simulink model "MCVT_Integration"  (R2020a)
settings: Solver=ode8  StartTime=0.0  StopTime=T_end  FixedStep=dt  RelTol=1e-3  EnableMultiTasking=on
values from neighbouring files (symbols this model uses):
  assumed_power_voltage = 1.25e2    (= 125)    [MCVT_Input_File.m]    (also in MCVT_Run_QuickCheck.m)
  battery_power_toggle = 1    [MCVT_Input_File.m]    (also in MCVT_Run_QuickCheck.m)
  battery_trickle_charge_param = 0.1    [MCVT_Input_File.m]    (also in MCVT_Run_QuickCheck.m)
  battery_trickle_leakage_param = 0.01    [MCVT_Input_File.m]    (also in MCVT_Run_QuickCheck.m)
  damage_case_id = 3    [MCVT_Input_File.m]    (also in MCVT_Run_QuickCheck.m = 1)
  dt = 1/fs    (= 0.001)    [MCVT_Run_QuickCheck.m]    (also in MCVT_Sim_Set.m)
  dt_2 = 1/fs_2    (= 0.001)    [MCVT_Run_QuickCheck.m]    (also in MCVT_Sim_Set.m)
  dt_3 = 1/fs_3    (= 0.001)    [MCVT_Run_QuickCheck.m]    (also in MCVT_Sim_Set.m)
  dt_5 = 1/fs_5    (= 0.005)    [MCVT_Run_QuickCheck.m]    (also in MCVT_Sim_Set.m)
  dt_8 = 1/fs_8    (= 0.05)    [MCVT_Run_QuickCheck.m]    (also in MCVT_Sim_Set.m)
  dt_9 = 1/fs_9    (= 0.001)    [MCVT_Run_QuickCheck.m]    (also in MCVT_Sim_Set.m)
  dust_met_duration = 3    [MCVT_Input_File.m]    (also in MCVT_Run_QuickCheck.m = 2)
  ECLSS_indoor_set_temperature = 298.15    [MCVT_Input_File.m]    (also in MCVT_Run_QuickCheck.m)
  energy_store_max_o = 150    [MCVT_Input_File.m]    (also in MCVT_Run_QuickCheck.m)
  energy_store_o = 120    [MCVT_Input_File.m]    (also in MCVT_Run_QuickCheck.m)
  fs = 1000    [MCVT_Run_QuickCheck.m]    (also in MCVT_Sim_Set.m)
  gain_charge_param = 1    [MCVT_Input_File.m]    (also in MCVT_Run_QuickCheck.m)
  ground_temperature = 373    [MCVT_Habitat_Design_Input_File.m]    (also in MCVT_Run_QuickCheck.m)
  I_max_charge = 800    [MCVT_Input_File.m]    (also in MCVT_Run_QuickCheck.m)
  I_max_discharge = 800    [MCVT_Input_File.m]    (also in MCVT_Run_QuickCheck.m)
  initial_pressure = 1e5    (= 100000)    [MCVT_Input_File.m]    (also in MCVT_Run_QuickCheck.m)
  initial_temperature = 295    [MCVT_Input_File.m]    (also in MCVT_Run_QuickCheck.m)
  interior_leak_rate = 0.00415    [MCVT_Input_File.m]    (also in MCVT_Run_QuickCheck.m)
  launch_dust_accum_start_time = 10    [MCVT_Input_File.m]    (also in MCVT_Run_QuickCheck.m = 2)
  launch_dust_accum_stop_time = 12    [MCVT_Input_File.m]    (also in MCVT_Run_QuickCheck.m = 3)
  meteorite_impact_time = 2    [MCVT_Input_File.m]    (also in MCVT_Run_QuickCheck.m = 1)
  nuclear_power_toggle = 1    [MCVT_Input_File.m]    (also in MCVT_Run_QuickCheck.m)
  print_interval = 30    [MCVT_Input_File.m]
  SOC_lower_thres = 0.5    [MCVT_Input_File.m]    (also in MCVT_Run_QuickCheck.m)
  SOC_upper_thres = 0.9    [MCVT_Input_File.m]    (also in MCVT_Run_QuickCheck.m)
  solar_power_toggle = 1    [MCVT_Input_File.m]    (also in MCVT_Run_QuickCheck.m)
  space_temperature = 4    [MCVT_Habitat_Design_Input_File.m]    (also in MCVT_Run_QuickCheck.m)
  Start_angle = 90    [MCVT_Input_File.m]    (also in MCVT_Run_QuickCheck.m)
undefined symbols (not defined in the file or in its folder): A_flow_restrictor, ACTIVITY_LOOKUP_TABLE, air, Aref, Bmap, Bmap2, Bmap3, comms, DiscIntNeverNeededParam, DmgSPL, dust_parameters, ECLSS_dust_damage_repair_time, ECLSS_heater_capacity, ECLSS_IPS_restrictor, ECLSS_IPS_setpointPressure, ECLSS_paint_damage_repair_time, ECLSS_radiatorLoop_discreteNumber, ECLSS_Vst, emptyActivity, expected_heat_add, FI, habitat, HIEM2SMM, HIEM2STM, HIEMDamageElements, impact_numbering, initial_SPLT_damage_index, input_air, input_Cond, input_CP, input_discretization, input_EV, input_Evap, input_radiatorLoop, K_ECLSS_dust_single, K_ECLSS_paint_single, K_Structure_damage_single, Kref, kW_to_W, meteoroidDustDep, mFRF, ndelays, nominalDustDep, noverlap, nuclear_toggle_gain, output_limits, panel_paint0, Ps0, reference_temperature, RepairTime_ECLSS_factor, Sensor_Location_SMM, SMM_T0, SMM_T1, SMM_T2, SMM_T3, SMM_T4, SMM_T4_0, SMM_T4_1, SMM_T4_2, solar_FDD_K ... (+73)
libraries: neural x350, simulink x39, xpcutilitieslib x7, xpcrtudplib x7, dspstat3 x1
(part 31 of 43)

== system MCVT_Integration/Sys2 - Structural System/Sys2_Structural_System/Fault Detection and Diagnosis (FDD) with Binary Classifer/Pattern Recognition Neural Network/Segment 5/Layer 2/Delays 1 ==
  "p{2}" : Inport  PortDimensions=20
      mask Display: e = 0.06;c1 = [120 230 180]/255;c2 = ([120 230 180]+0)/(256+128);c3 = ([120 230 180]+128)/(256+128);patch([0 1 1 0],[0 0 1 1],c1);patch([0 1 1 1-e 1-e 0+e],[0 0 1 1-e 0+e 0+e],c2);patch([0 0 1 1-e 0+e 0+e],[0 1 1 1-e 1-e 0+e],c3);plot([0 1 1 0 0],[0 0 1 1 0]);
  mux : Mux  Inputs=1
      mask Display: e = 0.06;c1 = [120 150 230]/255;c2 = ([120 150 230]+0)/383;c3 = ([120 150 230]+128)/383;patch([0 1 1 0],[0 0 1 1],c1);patch([0 1 1 1-e 1-e 0+e],[0 0 1 1-e 0+e 0+e],c2);patch([0 0 1 1-e 0+e 0+e],[0 1 1 1-e 1-e 0+e],c3);plot([0 1 1 0 0],[0 0 1 1 0]);disp('Mux')
  "pd{2,1}" : Outport
      mask Display: e = 0.06;c1 = [120 230 180]/255;c2 = ([120 230 180]+0)/(256+128);c3 = ([120 230 180]+128)/(256+128);patch([0 1 1 0],[0 0 1 1],c1);patch([0 1 1 1-e 1-e 0+e],[0 0 1 1-e 0+e 0+e],c2);patch([0 0 1 1-e 0+e 0+e],[0 1 1 1-e 1-e 0+e],c3);plot([0 1 1 0 0],[0 0 1 1 0]);
  wires:
  "p{2}"/1 -> mux/1
  mux/1 -> "pd{2,1}"/1

== system MCVT_Integration/Sys2 - Structural System/Sys2_Structural_System/Fault Detection and Diagnosis (FDD) with Binary Classifer/Pattern Recognition Neural Network/Segment 5/Layer 2/LW{2,1} ==
  "ad{2,1}" : Inport  PortDimensions=20
      mask Display: e = 0.06;c1 = [120 230 180]/255;c2 = ([120 230 180]+0)/(256+128);c3 = ([120 230 180]+128)/(256+128);patch([0 1 1 0],[0 0 1 1],c1);patch([0 1 1 1-e 1-e 0+e],[0 0 1 1-e 0+e 0+e],c2);patch([0 0 1 1-e 0+e 0+e],[0 1 1 1-e 1-e 0+e],c3);plot([0 1 1 0 0],[0 0 1 1 0]);
  "IW{2,1}(1,:)'" : Constant  Value=[-0.67773218447365179972763371551991440355777740478515625;0.29723131391808177426128168008290231227874755859375;-0.09310950608786626447876955126048414967954158782958984375;-0.25043409990960252553549025833490304648876190185546875;0.61520314771236161988099411246366798877716064453125;-0.4413810227549539444424908651853911578655242919921875;0.34640993668388009307790298407780937850475311279296875;0.58842174257296786521465037367306649684906005859375;-0.5081607293297121241693048432352952659130096435546875;0.033421296078495636716620964534740778617560863494873046875;0.496988773917953319259055433576577343046665191650390625;0.2455390127671049083080134778356296010315418243408203125;-0.224996153594978764989065211921115405857563018798828125;-0.3087985696736519347638250110321678221225738525390625;0.4009273835608577751798975441488437354564666748046875;-0.2431356717879919748792616474020178429782390594482421875;-0.00285707632105562832347533941401707124896347522735595703125;0.032728987946543490472617321529469336383044719696044921875;0.5245186178259164666570768531528301537036895751953125;-0.1546591117435880680108795104388264007866382598876953125]
      mask Display: e = 0.06;c1 = [120 150 230]/255;c2 = ([120 150 230]+0)/383;c3 = ([120 150 230]+128)/383;patch([0 1 1 0],[0 0 1 1],c1);patch([0 1 1 1-e 1-e 0+e],[0 0 1 1-e 0+e 0+e],c2);patch([0 0 1 1-e 0+e 0+e],[0 1 1 1-e 1-e 0+e],c3);plot([0 1 1 0 0],[0 0 1 1 0]);disp('weights');
  "IW{2,1}(10,:)'" : Constant  Value=[0.552549033571831937905471932026557624340057373046875;-0.09100490165966072797498753743639099411666393280029296875;0.1760541628457268081842101992151583544909954071044921875;0.5874239097493589856213702660170383751392364501953125;0.130139051658360982433038088856847025454044342041015625;0.5921645595936595452002393358270637691020965576171875;-0.06521020054191943582377888333212467841804027557373046875;0.28897880348058435640012930889497511088848114013671875;-0.1298384734383723204675931128804222680628299713134765625;-0.314572028887444898881398103185347281396389007568359375;0.65930167336349521089999825562699697911739349365234375;0.237364684824766303261611710695433430373668670654296875;-0.054096999788288357979837428501923568546772003173828125;0.51311436402968946257630022955709137022495269775390625;-0.55296838715594442970058253195020370185375213623046875;-0.1727245527295988292149075959969195537269115447998046875;-0.10587060479695453718562703215866349637508392333984375;-0.283737366362507958950089914651471190154552459716796875;0.26502997291755281406011590661364607512950897216796875;0.281267240887428382478674393496476113796234130859375]
      mask Display: e = 0.06;c1 = [120 150 230]/255;c2 = ([120 150 230]+0)/383;c3 = ([120 150 230]+128)/383;patch([0 1 1 0],[0 0 1 1],c1);patch([0 1 1 1-e 1-e 0+e],[0 0 1 1-e 0+e 0+e],c2);patch([0 0 1 1-e 0+e 0+e],[0 1 1 1-e 1-e 0+e],c3);plot([0 1 1 0 0],[0 0 1 1 0]);disp('weights');
  "IW{2,1}(11,:)'" : Constant  Value=[-0.05912874150283971286246043064238619990646839141845703125;0.54156082763547741532050849855295382440090179443359375;0.1892905576102281439698771237090113572776317596435546875;0.266587539549432428476194445465807802975177764892578125;0.2437897926806707749047831157440668903291225433349609375;-0.360503628753871208090231448295526206493377685546875;0.38970268980417788196035644432413391768932342529296875;0.01812511205154222759805549003431224264204502105712890625;-0.51079805029018710005317416289472021162509918212890625;0.331332206449338972920060086835292167961597442626953125;0.5113925621810591071181306688231416046619415283203125;-0.0743364509739538326105190435555414296686649322509765625;-0.088415771036461532350614334063720889389514923095703125;-0.5054613948697108671836986104608513414859771728515625;0.56960695088877766334434227246674709022045135498046875;-0.235268335550750384843610163443372584879398345947265625;0.4062060320473663832530064610182307660579681396484375;-0.4762276658674691187655980684212408959865570068359375;0.385169708871014704332225164762348867952823638916015625;-0.277613748702794704836804839942487888038158416748046875]
      mask Display: e = 0.06;c1 = [120 150 230]/255;c2 = ([120 150 230]+0)/383;c3 = ([120 150 230]+128)/383;patch([0 1 1 0],[0 0 1 1],c1);patch([0 1 1 1-e 1-e 0+e],[0 0 1 1-e 0+e 0+e],c2);patch([0 0 1 1-e 0+e 0+e],[0 1 1 1-e 1-e 0+e],c3);plot([0 1 1 0 0],[0 0 1 1 0]);disp('weights');
  "IW{2,1}(12,:)'" : Constant  Value=[0.43773075430955421527556836736039258539676666259765625;-0.0226631485883267702607923865798511542379856109619140625;0.1375752179635638672294817297370173037052154541015625;-0.342589307385872621214417677037999965250492095947265625;-0.00803372039310569323322663848330194014124572277069091796875;0.266163547138629719146507568439119495451450347900390625;0.0679141773643662516324326361427665688097476959228515625;0.447036832445153742288113107861136086285114288330078125;0.338363274166047001134671745603554882109165191650390625;0.63438020299558761649905136437155306339263916015625;0.4849211604715624179817723415908403694629669189453125;0.2307920076735069658813159776400425471365451812744140625;0.641689290985569815717326491721905767917633056640625;-0.61592841776821616317505458937375806272029876708984375;0.482357229715567437100531833493732847273349761962890625;-0.0080342268978742213680899197925100452266633510589599609375;0.299017768098594538539458653758629225194454193115234375;0.1865716668674242129100093734450638294219970703125;0.152140058661927202177110984848695807158946990966796875;-0.09721810243248285576811440478195436298847198486328125]
      mask Display: e = 0.06;c1 = [120 150 230]/255;c2 = ([120 150 230]+0)/383;c3 = ([120 150 230]+128)/383;patch([0 1 1 0],[0 0 1 1],c1);patch([0 1 1 1-e 1-e 0+e],[0 0 1 1-e 0+e 0+e],c2);patch([0 0 1 1-e 0+e 0+e],[0 1 1 1-e 1-e 0+e],c3);plot([0 1 1 0 0],[0 0 1 1 0]);disp('weights');
  "IW{2,1}(13,:)'" : Constant  Value=[0.4355595750049321424057779950089752674102783203125;-0.0895990004445349208506144123020931147038936614990234375;-0.54399989225422562544309812437859363853931427001953125;-0.373034500974306515441725196069455705583095550537109375;0.11066112771720294782884508322240435518324375152587890625;-0.3757367253088403913352522067725658416748046875;0.499029093144363000700280963428667746484279632568359375;-0.106510080176182408973062365475925616919994354248046875;0.320524922721690141980133148535969667136669158935546875;-0.16644989950893285257649267805390991270542144775390625;-0.035674542064537760610587469045640318654477596282958984375;-0.418057933762240152564260142753482796251773834228515625;-0.388561217554914739569227322135702706873416900634765625;0.13753317886975857664566547100548632442951202392578125;0.211068009211963392157684893390978686511516571044921875;0.51951396420677331544624166781431995332241058349609375;0.56988508309968899379072126976097933948040008544921875;0.481247016510891578722208805629634298384189605712890625;0.44357156438096811967852772795595228672027587890625;0.255785238287536575985114950526622124016284942626953125]
      mask Display: e = 0.06;c1 = [120 150 230]/255;c2 = ([120 150 230]+0)/383;c3 = ([120 150 230]+128)/383;patch([0 1 1 0],[0 0 1 1],c1);patch([0 1 1 1-e 1-e 0+e],[0 0 1 1-e 0+e 0+e],c2);patch([0 0 1 1-e 0+e 0+e],[0 1 1 1-e 1-e 0+e],c3);plot([0 1 1 0 0],[0 0 1 1 0]);disp('weights');
  "IW{2,1}(14,:)'" : Constant  Value=[-0.039786612181184351222373862810854916460812091827392578125;0.2321373358663718911021334179167752154171466827392578125;0.08032148343902671683292027182687888853251934051513671875;0.66783349466954777806648735349881462752819061279296875;0.0218740404012538088018846593740818207152187824249267578125;-0.284151817373596349280973072382039390504360198974609375;0.406206696173917414238729861608589999377727508544921875;-0.05018628210290389246583941940116346813738346099853515625;-0.111863721242385250054240941608441062271595001220703125;-0.59457414197112290477065243976539932191371917724609375;-0.00855983743492590154477994701665011234581470489501953125;-0.053293368746645163047670479272710508666932582855224609375;0.35542157490681181020164558503893204033374786376953125;0.5503721492865896181001517106778919696807861328125;-0.293494096559414108238428298136568628251552581787109375;-0.379628681256232669571915039341547526419162750244140625;-0.236013920704870983069412204713444225490093231201171875;0.47230602229098217481606525325332768261432647705078125;0.09948027948272873677293404170995927415788173675537109375;0.55264058309173236249733918157289735972881317138671875]
      mask Display: e = 0.06;c1 = [120 150 230]/255;c2 = ([120 150 230]+0)/383;c3 = ([120 150 230]+128)/383;patch([0 1 1 0],[0 0 1 1],c1);patch([0 1 1 1-e 1-e 0+e],[0 0 1 1-e 0+e 0+e],c2);patch([0 0 1 1-e 0+e 0+e],[0 1 1 1-e 1-e 0+e],c3);plot([0 1 1 0 0],[0 0 1 1 0]);disp('weights');
  "IW{2,1}(15,:)'" : Constant  Value=[0.1288107674958688664279549129787483252584934234619140625;-0.07497256937682771071873588653033948503434658050537109375;-0.30959773506689558519866523056407459080219268798828125;0.448215012435883630725896864532842300832271575927734375;-0.361768940527965099374085866656969301402568817138671875;-0.54297214933572546247120271800667978823184967041015625;0.054457371666344657479275070954827242530882358551025390625;0.125959936202742095456841298073413781821727752685546875;-0.2056921734268315204996468992248992435634136199951171875;0.440254257234804213805290373784373514354228973388671875;0.51034921534097066331270298178424127399921417236328125;0.468177463702322882443951357345213182270526885986328125;0.65075745752746716465964027520385570824146270751953125;-0.12131802595037254055565512089742696844041347503662109375;-0.42844264696869771569254226051270961761474609375;-0.323720290416319833592950772072072140872478485107421875;-0.064098745923206135177707665206980891525745391845703125;0.63669387601472882121100838048732839524745941162109375;-0.052305465433853150225562700370574020780622959136962890625;0.05665559541268076759745753179231542162597179412841796875]
      mask Display: e = 0.06;c1 = [120 150 230]/255;c2 = ([120 150 230]+0)/383;c3 = ([120 150 230]+128)/383;patch([0 1 1 0],[0 0 1 1],c1);patch([0 1 1 1-e 1-e 0+e],[0 0 1 1-e 0+e 0+e],c2);patch([0 0 1 1-e 0+e 0+e],[0 1 1 1-e 1-e 0+e],c3);plot([0 1 1 0 0],[0 0 1 1 0]);disp('weights');
  "IW{2,1}(16,:)'" : Constant  Value=[0.11737245423700033708502843410315108485519886016845703125;-0.27262057499895442358450736719532869756221771240234375;-0.07361864460219173789123914275478455238044261932373046875;-0.29779470754941239629687288470449857413768768310546875;0.386666490528341511012655473678023554384708404541015625;0.2476893163085038540582871746664750389754772186279296875;-0.6814743935251723616630670221638865768909454345703125;-0.59199523499185369157515879123820923268795013427734375;-0.299505833684180544640440757575561292469501495361328125;0.214060293872676610238414696141262538731098175048828125;-0.462623005264232833866344662965275347232818603515625;0.319710052566719860056565494232927449047565460205078125;-0.2728474794043964646306221766280941665172576904296875;0.265651446928187373597296527805156074464321136474609375;0.65459409742826590861142221910995431244373321533203125;-0.31617548578397947078855168001609854400157928466796875;-0.4149790189200015522175135629368014633655548095703125;-0.216229979661193283391185104846954345703125;-0.0700132160782936108223140081463498063385486602783203125;-0.29381327313157579173008571160607971251010894775390625]
      mask Display: e = 0.06;c1 = [120 150 230]/255;c2 = ([120 150 230]+0)/383;c3 = ([120 150 230]+128)/383;patch([0 1 1 0],[0 0 1 1],c1);patch([0 1 1 1-e 1-e 0+e],[0 0 1 1-e 0+e 0+e],c2);patch([0 0 1 1-e 0+e 0+e],[0 1 1 1-e 1-e 0+e],c3);plot([0 1 1 0 0],[0 0 1 1 0]);disp('weights');
  "IW{2,1}(17,:)'" : Constant  Value=[-0.65200183797321809020530736233922652900218963623046875;-0.513141636890571906093327925191260874271392822265625;0.6666627640293854017983221638132818043231964111328125;0.51126666389086794506368960355757735669612884521484375;0.142832758061001763305597478392883203923702239990234375;0.0309716089851164409763040197276495746336877346038818359375;-0.51906860495920581488604739206493832170963287353515625;0.455849206301122855666818622921709902584552764892578125;-0.5074134051478431661763579541002400219440460205078125;-0.483029589404079473524689092300832271575927734375;0.224793030869896603984869898340548388659954071044921875;0.168491582461909483736661741204443387687206268310546875;0.49576084119203611688675437108031474053859710693359375;-0.0518882463700796880967658353256410919129848480224609375;-0.458851615048450067746443892247043550014495849609375;0.1592196075605500638072697938696364872157573699951171875;-0.393400321923802642753997815816546790301799774169921875;0.06488101432483096842052106012488366104662418365478515625;-0.08832697515481234551426581447231001220643520355224609375;-0.310794878933715990054764688466093502938747406005859375]
      mask Display: e = 0.06;c1 = [120 150 230]/255;c2 = ([120 150 230]+0)/383;c3 = ([120 150 230]+128)/383;patch([0 1 1 0],[0 0 1 1],c1);patch([0 1 1 1-e 1-e 0+e],[0 0 1 1-e 0+e 0+e],c2);patch([0 0 1 1-e 0+e 0+e],[0 1 1 1-e 1-e 0+e],c3);plot([0 1 1 0 0],[0 0 1 1 0]);disp('weights');
  "IW{2,1}(18,:)'" : Constant  Value=[-0.58403843431543833730756887234747409820556640625;-0.11686344353476274537939616493531502783298492431640625;-0.208421829485184562091859561405726708471775054931640625;0.350835116678501368614462307959911413490772247314453125;-0.250053018739082866250811321151559241116046905517578125;-0.1767589718090601114131033000376191921532154083251953125;-0.6594792324061919952526977795059792697429656982421875;-0.2047483842336772219550056206571753136813640594482421875;0.0799817324020934972139684759895317256450653076171875;-0.263873314132044234359142365065054036676883697509765625;0.1238058615990984823174159146219608373939990997314453125;-0.2427010547863654721378878775794873945415019989013671875;-0.340912365339598844027335644568665884435176849365234375;-0.40629502196977806960376256029121577739715576171875;0.05821085493265502897042296126528526656329631805419921875;-0.4923309925498069450355842491262592375278472900390625;-0.63697077823032144383574859602958895266056060791015625;0.70272777928235630184872206882573664188385009765625;0.082408225308850002743810136962565593421459197998046875;0.257126723858133787725677166235982440412044525146484375]
      mask Display: e = 0.06;c1 = [120 150 230]/255;c2 = ([120 150 230]+0)/383;c3 = ([120 150 230]+128)/383;patch([0 1 1 0],[0 0 1 1],c1);patch([0 1 1 1-e 1-e 0+e],[0 0 1 1-e 0+e 0+e],c2);patch([0 0 1 1-e 0+e 0+e],[0 1 1 1-e 1-e 0+e],c3);plot([0 1 1 0 0],[0 0 1 1 0]);disp('weights');
  "IW{2,1}(19,:)'" : Constant  Value=[0.66085390281812073087763792500481940805912017822265625;-0.4135361056779769395319590330473147332668304443359375;0.1428003055410909383216022661144961602985858917236328125;0.39212017801967302688126437715254724025726318359375;-0.5914533321008648147909525505383498966693878173828125;0.468354086242417422258910164600820280611515045166015625;0.33818694482531197476049555916688404977321624755859375;-0.10651793410114475391115007596454233862459659576416015625;0.01404438680674700845596447607022128067910671234130859375;-0.067871504435023599643983516216394491493701934814453125;-0.58546404149150454987449165855650790035724639892578125;-0.24986074727083884550182801831397227942943572998046875;-0.33108516389830178194841892036492936313152313232421875;0.1009772519076451990915899159517721273005008697509765625;-0.2363686013993024082058269641493097878992557525634765625;-0.62080475837335746813749892680789344012737274169921875;0.25979730822451652105797847980284132063388824462890625;-0.31588222351298089751736597463604994118213653564453125;-0.449657863213273056857133269659243524074554443359375;0.48004422581746719611572871144744567573070526123046875]
      mask Display: e = 0.06;c1 = [120 150 230]/255;c2 = ([120 150 230]+0)/383;c3 = ([120 150 230]+128)/383;patch([0 1 1 0],[0 0 1 1],c1);patch([0 1 1 1-e 1-e 0+e],[0 0 1 1-e 0+e 0+e],c2);patch([0 0 1 1-e 0+e 0+e],[0 1 1 1-e 1-e 0+e],c3);plot([0 1 1 0 0],[0 0 1 1 0]);disp('weights');
  "IW{2,1}(2,:)'" : Constant  Value=[0.264866509139968731023628834009286947548389434814453125;-0.421276202799477472726863425123156048357486724853515625;-0.32172884927020184431256666357512585818767547607421875;0.0873332852793348435316289624097407795488834381103515625;0.45725062710630892670593539151013828814029693603515625;-0.47027317103559440614191089480300433933734893798828125;0.50420051952175215514984074616222642362117767333984375;0.2021495935450947911693475589345325715839862823486328125;0.5958119042887479732684141708887182176113128662109375;0.549176439745886479926184620126150548458099365234375;-0.07382040642580724176813333770041936077177524566650390625;-0.0911008824706815711902407883826526813209056854248046875;0.472789640540149047609475019271485507488250732421875;-0.302487138214584894146952365190372802317142486572265625;0.13705931754781619513750001715379767119884490966796875;0.02691598569268426055511866934466524980962276458740234375;-0.61260682814079270297469292927416972815990447998046875;0.212242740676705288205283750357921235263347625732421875;-0.45586121041979865697868490315158851444721221923828125;-0.173654441390307334724951715543284080922603607177734375]
      mask Display: e = 0.06;c1 = [120 150 230]/255;c2 = ([120 150 230]+0)/383;c3 = ([120 150 230]+128)/383;patch([0 1 1 0],[0 0 1 1],c1);patch([0 1 1 1-e 1-e 0+e],[0 0 1 1-e 0+e 0+e],c2);patch([0 0 1 1-e 0+e 0+e],[0 1 1 1-e 1-e 0+e],c3);plot([0 1 1 0 0],[0 0 1 1 0]);disp('weights');
  "IW{2,1}(20,:)'" : Constant  Value=[-0.4175468971215832159060710182529874145984649658203125;0.03969986159828763072621171659193350933492183685302734375;-0.1566172687200387547701296853119856677949428558349609375;-0.32922271339706554726944887079298496246337890625;-0.51801946227475414996632707698154263198375701904296875;-0.307456855608206625074529938501655124127864837646484375;0.384731736291375525649272049122373573482036590576171875;0.04812249993952956439446921876879059709608554840087890625;-0.090638927458235374334805101170786656439304351806640625;0.122542975183939584216119556003832258284091949462890625;-0.29383378097437728992957772788940928876399993896484375;0.438785700581473037118485081009566783905029296875;-0.399251041064929090840252001726184971630573272705078125;0.35533599456226572232964144859579391777515411376953125;0.4899082557321496889102263594395481050014495849609375;-0.597079247906687982094808830879628658294677734375;0.304628463384683134673736049080616794526576995849609375;-0.343082762765438975538501154005643911659717559814453125;-0.512278720367670192814557594829238951206207275390625;0.2101428795036640873039601729033165611326694488525390625]
      mask Display: e = 0.06;c1 = [120 150 230]/255;c2 = ([120 150 230]+0)/383;c3 = ([120 150 230]+128)/383;patch([0 1 1 0],[0 0 1 1],c1);patch([0 1 1 1-e 1-e 0+e],[0 0 1 1-e 0+e 0+e],c2);patch([0 0 1 1-e 0+e 0+e],[0 1 1 1-e 1-e 0+e],c3);plot([0 1 1 0 0],[0 0 1 1 0]);disp('weights');
  "IW{2,1}(3,:)'" : Constant  Value=[-0.63641337529254382587851068819873034954071044921875;-0.0538200760248459253443087391133303754031658172607421875;0.5922950469448557964113888374413363635540008544921875;0.1104467021817938654493218564311973750591278076171875;0.1806035590923620592906928550291922874748706817626953125;0.155887215757943675686902906818431802093982696533203125;0.54948418132535004065886141688679344952106475830078125;-0.29211408337009847624443636959767900407314300537109375;-0.3147777442644297263285579902003519237041473388671875;-0.69197722481291512597323389854864217340946197509765625;-0.057506131833469857628227117629649001173675060272216796875;-0.0458575524505141218778447864679037593305110931396484375;0.466154700686250311747471641865558922290802001953125;-0.56359055298359728158885673110489733517169952392578125;0.448293072793023494870823242308688350021839141845703125;-0.338786234188482471285652763981488533318042755126953125;0.10964982306623523300448397321815718896687030792236328125;-0.5683152258332595518908192389062605798244476318359375;-0.0799660657061919966093199718670803122222423553466796875;0.291955469599589179097165470011532306671142578125]
      mask Display: e = 0.06;c1 = [120 150 230]/255;c2 = ([120 150 230]+0)/383;c3 = ([120 150 230]+128)/383;patch([0 1 1 0],[0 0 1 1],c1);patch([0 1 1 1-e 1-e 0+e],[0 0 1 1-e 0+e 0+e],c2);patch([0 0 1 1-e 0+e 0+e],[0 1 1 1-e 1-e 0+e],c3);plot([0 1 1 0 0],[0 0 1 1 0]);disp('weights');
  "IW{2,1}(4,:)'" : Constant  Value=[-0.06798263044347004069312134788560797460377216339111328125;-0.0186539241920614003167333549981776741333305835723876953125;0.1963568907594429313601125386412604711949825286865234375;-0.278086006373269245539603389261174015700817108154296875;0.50344393773321571838863519587903283536434173583984375;-0.490684781110136969406454454656341113150119781494140625;-0.256625611759414506973797642785939387977123260498046875;-0.325909728784971874926412738204817287623882293701171875;-0.5519555598535295093398644894477911293506622314453125;0.4112062769324780209245773221482522785663604736328125;0.21641988860370975356062217542785219848155975341796875;0.52950061641745971297012829381856136023998260498046875;-0.298066083811056425911800715766730718314647674560546875;-0.400971735467876289060740191416698507964611053466796875;-0.343856983708815011624437829595990478992462158203125;0.421422153423563095042680970436776988208293914794921875;0.110638280100366637181963369584991596639156341552734375;-0.0006762035295504336719629723262414700002409517765045166015625;-0.5013195868569371160816672272630967199802398681640625;0.52438242058926476385494197529624216258525848388671875]
      mask Display: e = 0.06;c1 = [120 150 230]/255;c2 = ([120 150 230]+0)/383;c3 = ([120 150 230]+128)/383;patch([0 1 1 0],[0 0 1 1],c1);patch([0 1 1 1-e 1-e 0+e],[0 0 1 1-e 0+e 0+e],c2);patch([0 0 1 1-e 0+e 0+e],[0 1 1 1-e 1-e 0+e],c3);plot([0 1 1 0 0],[0 0 1 1 0]);disp('weights');
  "IW{2,1}(5,:)'" : Constant  Value=[0.439957103977431096542005661831353791058063507080078125;-0.32791611699534717327964017385966144502162933349609375;0.134094223101226817096431886966456659138202667236328125;0.1181619493190914915725642231336678378283977508544921875;0.4862867358141655937942005039076320827007293701171875;0.394529198406466774518008833183557726442813873291015625;0.63198247203318114184611431483062915503978729248046875;-0.59072823194747547947969223969266749918460845947265625;-0.405994287272546661160532721623894758522510528564453125;-0.007982490219878797532704339801057358272373676300048828125;-0.157408378342908938929411988283391110599040985107421875;0.366819631921708466659737268855678848922252655029296875;0.0246784029376982093351156066773910424672067165374755859375;-0.30044146293516604817597226428915746510028839111328125;0.0300721220874937238132229566645037266425788402557373046875;0.10520071890439909589876066320357494987547397613525390625;-0.09913541437868196581906232722758431918919086456298828125;-0.50632363527212975728986066314973868429660797119140625;-0.69380483998050268557022945969947613775730133056640625;-0.276804910890988242755383907933719456195831298828125]
      mask Display: e = 0.06;c1 = [120 150 230]/255;c2 = ([120 150 230]+0)/383;c3 = ([120 150 230]+128)/383;patch([0 1 1 0],[0 0 1 1],c1);patch([0 1 1 1-e 1-e 0+e],[0 0 1 1-e 0+e 0+e],c2);patch([0 0 1 1-e 0+e 0+e],[0 1 1 1-e 1-e 0+e],c3);plot([0 1 1 0 0],[0 0 1 1 0]);disp('weights');
  "IW{2,1}(6,:)'" : Constant  Value=[-0.05661177237957155938818942786383558996021747589111328125;0.044211969975700600443691001828483422286808490753173828125;-0.7074463809755455745431618197471834719181060791015625;-0.1658840422485735877078383282423601485788822174072265625;0.474683209522257343326856471321661956608295440673828125;-0.19065295525132264220502520402078516781330108642578125;0.2331535575113192482188395615594345144927501678466796875;0.57692282892692003937185063477954827249050140380859375;0.28335335720208032483924398547969758510589599609375;-0.42976821892095562471780567648238502442836761474609375;0.0072096388509773241815015154543289099819958209991455078125;0.33368066595460543766193950432352721691131591796875;-0.01141511104865122749885131270275451242923736572265625;0.383727527807560309103251938722678460180759429931640625;-0.13548382201343944775118188772466965019702911376953125;0.4549201798342981106770821497775614261627197265625;0.2978292482891584658233341542654670774936676025390625;-0.2392796951385826320635175079587497748434543609619140625;-0.589039014937933647075851695262826979160308837890625;-0.47861095923085417158659993219771422445774078369140625]
      mask Display: e = 0.06;c1 = [120 150 230]/255;c2 = ([120 150 230]+0)/383;c3 = ([120 150 230]+128)/383;patch([0 1 1 0],[0 0 1 1],c1);patch([0 1 1 1-e 1-e 0+e],[0 0 1 1-e 0+e 0+e],c2);patch([0 0 1 1-e 0+e 0+e],[0 1 1 1-e 1-e 0+e],c3);plot([0 1 1 0 0],[0 0 1 1 0]);disp('weights');
  "IW{2,1}(7,:)'" : Constant  Value=[-0.1872712372599601715617012587244971655309200286865234375;0.09837392502695159468562025040228036232292652130126953125;0.46203343810884900388913365532062016427516937255859375;0.53665625840559705306276327974046580493450164794921875;0.423852317842340509113086000070325098931789398193359375;-0.56560679653847711900738204349181614816188812255859375;-0.562857769996844670146174394176341593265533447265625;0.050873402525171827759375986488521448336541652679443359375;0.389865226961422062235129715190851129591464996337890625;0.5986237036808181866121003622538410127162933349609375;0.040942220676205509644063340601860545575618743896484375;-0.0206999186992972393495104910243753693066537380218505859375;0.058385556967571881903378283595884568057954311370849609375;0.2270565758070160755632826976579963229596614837646484375;-0.46909440009930902792945062174112536013126373291015625;0.57384001049193422705485545520787127315998077392578125;0.1963272237354528881869697443107725121080875396728515625;-0.165453422961946372549135730878333561122417449951171875;0.23171417063125054891514764676685445010662078857421875;-0.1681114484372078499152536323890672065317630767822265625]
      mask Display: e = 0.06;c1 = [120 150 230]/255;c2 = ([120 150 230]+0)/383;c3 = ([120 150 230]+128)/383;patch([0 1 1 0],[0 0 1 1],c1);patch([0 1 1 1-e 1-e 0+e],[0 0 1 1-e 0+e 0+e],c2);patch([0 0 1 1-e 0+e 0+e],[0 1 1 1-e 1-e 0+e],c3);plot([0 1 1 0 0],[0 0 1 1 0]);disp('weights');
  "IW{2,1}(8,:)'" : Constant  Value=[0.45592763811386038952377930399961769580841064453125;0.057143436805770032871354402459473931230604648590087890625;0.1351215776388423328580046245406265370547771453857421875;-0.10685055870610761508121555607431218959391117095947265625;-0.100986430894486167719747982118860818445682525634765625;-0.278723051569358337697934757670736871659755706787109375;0.34237487434339897163226851262152194976806640625;0.51541155122957571510511343149119056761264801025390625;-0.486499896428013223204089854334597475826740264892578125;0.33444133702258194151824000073247589170932769775390625;-0.30790705310735921873543929905281402170658111572265625;0.1367464650132592385300966952854651026427745819091796875;-0.57642887893386862696587513710255734622478485107421875;-0.2036191832959350633824868737065116874873638153076171875;0.4030383487701107991796334317768923938274383544921875;-0.48667903489693353780154438936733640730381011962890625;0.395884101990914893942630214951350353658199310302734375;0.57190767488089011560958851987379603087902069091796875;0.25761351467509785440057612504460848867893218994140625;-0.305143967145103667260030988472863100469112396240234375]
      mask Display: e = 0.06;c1 = [120 150 230]/255;c2 = ([120 150 230]+0)/383;c3 = ([120 150 230]+128)/383;patch([0 1 1 0],[0 0 1 1],c1);patch([0 1 1 1-e 1-e 0+e],[0 0 1 1-e 0+e 0+e],c2);patch([0 0 1 1-e 0+e 0+e],[0 1 1 1-e 1-e 0+e],c3);plot([0 1 1 0 0],[0 0 1 1 0]);disp('weights');
  "IW{2,1}(9,:)'" : Constant  Value=[-0.186118037795272417422864918989944271743297576904296875;-0.22114103093471870753461416825302876532077789306640625;0.42123896788813464109324513628962449729442596435546875;0.57649205720862328750087044682004489004611968994140625;-0.28421094992462248018938453242299146950244903564453125;0.34621367724795815146165978148928843438625335693359375;0.291378454104244510869392570384661667048931121826171875;-0.5750754489244744860343416803516447544097900390625;0.2567611368671796068241519606090150773525238037109375;0.2749163796331861586708100730902515351772308349609375;0.2825771857085357385841462019016034901142120361328125;0.3689238321454257896192530097323469817638397216796875;0.297418373264197455352331189715187065303325653076171875;0.27165302730286089438749286273377947509288787841796875;-0.1056564013032067206321329422280541621148586273193359375;0.0987404660911136156098422134164138697087764739990234375;0.5144519005348289208967571539687924087047576904296875;0.498414591291945152651265971144312061369419097900390625;0.7219280227342921296695976707269437611103057861328125;0.099696984939066923825379262780188582837581634521484375]
      mask Display: e = 0.06;c1 = [120 150 230]/255;c2 = ([120 150 230]+0)/383;c3 = ([120 150 230]+128)/383;patch([0 1 1 0],[0 0 1 1],c1);patch([0 1 1 1-e 1-e 0+e],[0 0 1 1-e 0+e 0+e],c2);patch([0 0 1 1-e 0+e 0+e],[0 1 1 1-e 1-e 0+e],c3);plot([0 1 1 0 0],[0 0 1 1 0]);disp('weights');
  Mux : Mux  Inputs=20
      mask Display: e = 0.06;c1 = [120 150 230]/255;c2 = ([120 150 230]+0)/383;c3 = ([120 150 230]+128)/383;patch([0 1 1 0],[0 0 1 1],c1);patch([0 1 1 1-e 1-e 0+e],[0 0 1 1-e 0+e 0+e],c2);patch([0 0 1 1-e 0+e 0+e],[0 1 1 1-e 1-e 0+e],c3);plot([0 1 1 0 0],[0 0 1 1 0]);disp('Mux');
  dotprod{1..20} (20 alike) : neural/Weight Functions/dotprod
  "lz{2,1}" : Outport
      mask Display: e = 0.06;c1 = [120 230 180]/255;c2 = ([120 230 180]+0)/(256+128);c3 = ([120 230 180]+128)/(256+128);patch([0 1 1 0],[0 0 1 1],c1);patch([0 1 1 1-e 1-e 0+e],[0 0 1 1-e 0+e 0+e],c2);patch([0 0 1 1-e 0+e 0+e],[0 1 1 1-e 1-e 0+e],c3);plot([0 1 1 0 0],[0 0 1 1 0]);
  wires:
  "IW{2,1}(1,:)'"/1 -> dotprod1/1
  "ad{2,1}"/1 -> dotprod1/2, dotprod2/2, dotprod3/2, dotprod4/2, dotprod5/2, dotprod6/2, dotprod7/2, dotprod8/2, dotprod9/2, dotprod10/2, dotprod11/2, dotprod12/2, dotprod13/2, dotprod14/2, dotprod15/2, dotprod16/2, dotprod17/2, dotprod18/2, dotprod19/2, dotprod20/2
  dotprod1/1 -> Mux/1
  "IW{2,1}(2,:)'"/1 -> dotprod2/1
  dotprod2/1 -> Mux/2
  "IW{2,1}(3,:)'"/1 -> dotprod3/1
  dotprod3/1 -> Mux/3
  "IW{2,1}(4,:)'"/1 -> dotprod4/1
  dotprod4/1 -> Mux/4
  "IW{2,1}(5,:)'"/1 -> dotprod5/1
  dotprod5/1 -> Mux/5
  "IW{2,1}(6,:)'"/1 -> dotprod6/1
  dotprod6/1 -> Mux/6
  "IW{2,1}(7,:)'"/1 -> dotprod7/1
  dotprod7/1 -> Mux/7
  "IW{2,1}(8,:)'"/1 -> dotprod8/1
  dotprod8/1 -> Mux/8
  "IW{2,1}(9,:)'"/1 -> dotprod9/1
  dotprod9/1 -> Mux/9
  "IW{2,1}(10,:)'"/1 -> dotprod10/1
  dotprod10/1 -> Mux/10
  "IW{2,1}(11,:)'"/1 -> dotprod11/1
  dotprod11/1 -> Mux/11
  "IW{2,1}(12,:)'"/1 -> dotprod12/1
  dotprod12/1 -> Mux/12
  "IW{2,1}(13,:)'"/1 -> dotprod13/1
  dotprod13/1 -> Mux/13
  "IW{2,1}(14,:)'"/1 -> dotprod14/1
  dotprod14/1 -> Mux/14
  "IW{2,1}(15,:)'"/1 -> dotprod15/1
  dotprod15/1 -> Mux/15
  "IW{2,1}(16,:)'"/1 -> dotprod16/1
  dotprod16/1 -> Mux/16
  "IW{2,1}(17,:)'"/1 -> dotprod17/1
  dotprod17/1 -> Mux/17
  "IW{2,1}(18,:)'"/1 -> dotprod18/1
  dotprod18/1 -> Mux/18
  "IW{2,1}(19,:)'"/1 -> dotprod19/1
  dotprod19/1 -> Mux/19
  "IW{2,1}(20,:)'"/1 -> dotprod20/1
  dotprod20/1 -> Mux/20
  Mux/1 -> "lz{2,1}"/1

== system MCVT_Integration/Sys2 - Structural System/Sys2_Structural_System/Fault Detection and Diagnosis (FDD) with Binary Classifer/Pattern Recognition Neural Network/Segment 5/Layer 3/Delays 1 ==
  "p{3}" : Inport  PortDimensions=20
      mask Display: e = 0.06;c1 = [120 230 180]/255;c2 = ([120 230 180]+0)/(256+128);c3 = ([120 230 180]+128)/(256+128);patch([0 1 1 0],[0 0 1 1],c1);patch([0 1 1 1-e 1-e 0+e],[0 0 1 1-e 0+e 0+e],c2);patch([0 0 1 1-e 0+e 0+e],[0 1 1 1-e 1-e 0+e],c3);plot([0 1 1 0 0],[0 0 1 1 0]);
  mux : Mux  Inputs=1
      mask Display: e = 0.06;c1 = [120 150 230]/255;c2 = ([120 150 230]+0)/383;c3 = ([120 150 230]+128)/383;patch([0 1 1 0],[0 0 1 1],c1);patch([0 1 1 1-e 1-e 0+e],[0 0 1 1-e 0+e 0+e],c2);patch([0 0 1 1-e 0+e 0+e],[0 1 1 1-e 1-e 0+e],c3);plot([0 1 1 0 0],[0 0 1 1 0]);disp('Mux')
  "pd{3,2}" : Outport
      mask Display: e = 0.06;c1 = [120 230 180]/255;c2 = ([120 230 180]+0)/(256+128);c3 = ([120 230 180]+128)/(256+128);patch([0 1 1 0],[0 0 1 1],c1);patch([0 1 1 1-e 1-e 0+e],[0 0 1 1-e 0+e 0+e],c2);patch([0 0 1 1-e 0+e 0+e],[0 1 1 1-e 1-e 0+e],c3);plot([0 1 1 0 0],[0 0 1 1 0]);
  wires:
  "p{3}"/1 -> mux/1
  mux/1 -> "pd{3,2}"/1

== system MCVT_Integration/Sys2 - Structural System/Sys2_Structural_System/Fault Detection and Diagnosis (FDD) with Binary Classifer/Pattern Recognition Neural Network/Segment 5/Layer 3/LW{3,2} ==
  "ad{3,2}" : Inport  PortDimensions=20
      mask Display: e = 0.06;c1 = [120 230 180]/255;c2 = ([120 230 180]+0)/(256+128);c3 = ([120 230 180]+128)/(256+128);patch([0 1 1 0],[0 0 1 1],c1);patch([0 1 1 1-e 1-e 0+e],[0 0 1 1-e 0+e 0+e],c2);patch([0 0 1 1-e 0+e 0+e],[0 1 1 1-e 1-e 0+e],c3);plot([0 1 1 0 0],[0 0 1 1 0]);
  "IW{3,2}(1,:)'" : Constant  Value=[-0.28170146099803206052314408225356601178646087646484375;-0.76120722780956617814496212304220534861087799072265625;0.8504712828044385108938740813755430281162261962890625;-0.9586125379225769460589390291715972125530242919921875;0.126374771183149270381562701004440896213054656982421875;-0.59985424625831818001842066223616711795330047607421875;0.56413511735130039692620584901305846869945526123046875;-0.041940446655469441894492632627589046023786067962646484375;0.56939399945986668427622134913690388202667236328125;0.85776646553798718830563529991195537149906158447265625;0.46976595114895669524912591441534459590911865234375;0.180223574341127179554433723751571960747241973876953125;-0.385050267995992012703965201581013388931751251220703125;0.66601814917987056663406519874115474522113800048828125;-0.925599617386438477950605374644510447978973388671875;-0.73399059566546898114580699257203377783298492431640625;1.229448970258027795665611847653053700923919677734375;0.2228497751183370623184742953526438213884830474853515625;-0.679625555142887805004647816531360149383544921875;-0.1165111396264187637594744728630757890641689300537109375]
      mask Display: e = 0.06;c1 = [120 150 230]/255;c2 = ([120 150 230]+0)/383;c3 = ([120 150 230]+128)/383;patch([0 1 1 0],[0 0 1 1],c1);patch([0 1 1 1-e 1-e 0+e],[0 0 1 1-e 0+e 0+e],c2);patch([0 0 1 1-e 0+e 0+e],[0 1 1 1-e 1-e 0+e],c3);plot([0 1 1 0 0],[0 0 1 1 0]);disp('weights');
  "IW{3,2}(2,:)'" : Constant  Value=[-1.0004490279308730915630576419061981141567230224609375;-0.2184955957196986486845702302161953411996364593505859375;-0.448518133213831771133328629730385728180408477783203125;-0.452706748670462955086435385965160094201564788818359375;0.91399017908161950263234984959126450121402740478515625;0.0751440200134909364049207169955479912459850311279296875;0.64586194386665407574099617704632692039012908935546875;0.6995981021734889448993044425151310861110687255859375;-0.1735635904975613430512026980068185366690158843994140625;-0.232563387873075566592007135113817639648914337158203125;0.50600196399877417352541897344053722918033599853515625;0.288760814654013098223828137633972801268100738525390625;-0.10487781591015947479927916674569132737815380096435546875;-0.96544906608476999121393191671813838183879852294921875;-0.62934931692248097245823146295151673257350921630859375;-0.85021028999218761779133046729839406907558441162109375;-0.8496389522221063916873617927194572985172271728515625;-0.27243681397338803673591201004455797374248504638671875;0.49429781055168942049959923679125495254993438720703125;0.85792424733700867189867267370573244988918304443359375]
      mask Display: e = 0.06;c1 = [120 150 230]/255;c2 = ([120 150 230]+0)/383;c3 = ([120 150 230]+128)/383;patch([0 1 1 0],[0 0 1 1],c1);patch([0 1 1 1-e 1-e 0+e],[0 0 1 1-e 0+e 0+e],c2);patch([0 0 1 1-e 0+e 0+e],[0 1 1 1-e 1-e 0+e],c3);plot([0 1 1 0 0],[0 0 1 1 0]);disp('weights');
  Mux : Mux  Inputs=2
      mask Display: e = 0.06;c1 = [120 150 230]/255;c2 = ([120 150 230]+0)/383;c3 = ([120 150 230]+128)/383;patch([0 1 1 0],[0 0 1 1],c1);patch([0 1 1 1-e 1-e 0+e],[0 0 1 1-e 0+e 0+e],c2);patch([0 0 1 1-e 0+e 0+e],[0 1 1 1-e 1-e 0+e],c3);plot([0 1 1 0 0],[0 0 1 1 0]);disp('Mux');
  dotprod1 : neural/Weight Functions/dotprod
  dotprod2 : neural/Weight Functions/dotprod
  "lz{3,2}" : Outport
      mask Display: e = 0.06;c1 = [120 230 180]/255;c2 = ([120 230 180]+0)/(256+128);c3 = ([120 230 180]+128)/(256+128);patch([0 1 1 0],[0 0 1 1],c1);patch([0 1 1 1-e 1-e 0+e],[0 0 1 1-e 0+e 0+e],c2);patch([0 0 1 1-e 0+e 0+e],[0 1 1 1-e 1-e 0+e],c3);plot([0 1 1 0 0],[0 0 1 1 0]);
  wires:
  "IW{3,2}(1,:)'"/1 -> dotprod1/1
  "ad{3,2}"/1 -> dotprod1/2, dotprod2/2
  dotprod1/1 -> Mux/1
  "IW{3,2}(2,:)'"/1 -> dotprod2/1
  dotprod2/1 -> Mux/2
  Mux/1 -> "lz{3,2}"/1

== system MCVT_Integration/Sys2 - Structural System/Sys2_Structural_System/Fault Detection and Diagnosis (FDD) with Binary Classifer/Subsystem/Enabled Subsystem3/Pattern Recognition Neural Network1/Layer 1 ==
  "p{1}" : Inport  PortDimensions=45  SampleTime=0.001
      mask Display: e = 0.06;c1 = [120 230 180]/255;c2 = ([120 230 180]+0)/(256+128);c3 = ([120 230 180]+128)/(256+128);patch([0 1 1 0],[0 0 1 1],c1);patch([0 1 1 1-e 1-e 0+e],[0 0 1 1-e 0+e 0+e],c2);patch([0 0 1 1-e 0+e 0+e],[0 1 1 1-e 1-e 0+e],c3);plot([0 1 1 0 0],[0 0 1 1 0]);
  Delays 1 : Subsystem
      mask Display: e = 0.06;c1 = [120 150 230]/255;c2 = ([120 150 230]+0)/383;c3 = ([120 150 230]+128)/383;patch([0 1 1 0],[0 0 1 1],c1);patch([0 1 1 1-e 1-e 0+e],[0 0 1 1-e 0+e 0+e],c2);patch([0 0 1 1-e 0+e 0+e],[0 1 1 1-e 1-e 0+e],c3);plot([0 1 1 0 0],[0 0 1 1 0]);disp('0');
  "IW{1,1}" : Subsystem
      mask Display: e = 0.06;c1 = [120 150 230]/255;c2 = ([120 150 230]+0)/383;c3 = ([120 150 230]+128)/383;patch([0 1 1 0],[0 0 1 1],c1);patch([0 1 1 1-e 1-e 0+e],[0 0 1 1-e 0+e 0+e],c2);patch([0 0 1 1-e 0+e 0+e],[0 1 1 1-e 1-e 0+e],c3);plot([0 1 1 0 0],[0 0 1 1 0]);disp('W');
  "b{1}" : Constant  Value=[-1.5256133296022216239151703121024183928966522216796875;-1.340419889257152608053047515568323433399200439453125;-1.277059131681266279656483675353229045867919921875;-1.2288647856494863130905059733777306973934173583984375;1.0966929016127273666114660954917781054973602294921875;0.99012431554484459095277770757093094289302825927734375;-0.83385649693403995907914350027567707002162933349609375;-0.71671461105535072544370223113219253718852996826171875;-0.7809321027937332626578381677973084151744842529296875;-0.5030235408363574745038704350008629262447357177734375;-0.374885107127588768083370496242423541843891143798828125;0.30670454139929803627495630280463956296443939208984375;-0.293228033582005831458872080474975518882274627685546875;0.114639663734024621621898631929070688784122467041015625;-0.061570795253310016403869298073914251290261745452880859375;-0.113416249637178501163958799224928952753543853759765625;0.0262107307592271028540142907559129525907337665557861328125;0.2317446592113069636642563864370458759367465972900390625;-0.447035472110449749738592117864754982292652130126953125;-0.52155339637859288171028993019717745482921600341796875;0.5479047708225810442428382884827442467212677001953125;-0.62836902694331320251563965939567424356937408447265625;-0.80147388269306085728516109156771562993526458740234375;-0.8642109150275363305127029889263212680816650390625;0.921457768094346985066067645675502717494964599609375;1.0019238402761103667870656863669864833354949951171875;1.1920495454348845054681760302628390491008758544921875;-1.363891380039423228964778900262899696826934814453125;1.41113568903686559252719234791584312915802001953125;-1.4675910351675494069922933704219758510589599609375]
      mask Display: e = 0.06;c1 = [120 150 230]/255;c2 = ([120 150 230]+0)/383;c3 = ([120 150 230]+128)/383;patch([0 1 1 0],[0 0 1 1],c1);patch([0 1 1 1-e 1-e 0+e],[0 0 1 1-e 0+e 0+e],c2);patch([0 0 1 1-e 0+e 0+e],[0 1 1 1-e 1-e 0+e],c3);plot([0 1 1 0 0],[0 0 1 1 0]);disp('b');
  netsum : Sum  Inputs=2  InputSameDT=on  SaturateOnIntegerOverflow=on  OutDataTypeStr=Inherit: Same as first input
      mask Display: e = 0.06;c1 = [120 150 230]/255;c2 = ([120 150 230]+0)/383;c3 = ([120 150 230]+128)/383;patch([0 1 1 0],[0 0 1 1],c1);patch([0 1 1 1-e 1-e 0+e],[0 0 1 1-e 0+e 0+e],c2);patch([0 0 1 1-e 0+e 0+e],[0 1 1 1-e 1-e 0+e],c3);plot([0 1 1 0 0],[0 0 1 1 0]);disp('+');
  tansig : neural/Transfer Functions/tansig
  "a{1}" : Outport  InitialOutput=[0;0;0;0;0;0;0;0;0;0;0;0;0;0;0;0;0;0;0;0;0;0;0;0;0;0;0;0;0;0]
      mask Display: e = 0.06;c1 = [120 230 180]/255;c2 = ([120 230 180]+0)/(256+128);c3 = ([120 230 180]+128)/(256+128);patch([0 1 1 0],[0 0 1 1],c1);patch([0 1 1 1-e 1-e 0+e],[0 0 1 1-e 0+e 0+e],c2);patch([0 0 1 1-e 0+e 0+e],[0 1 1 1-e 1-e 0+e],c3);plot([0 1 1 0 0],[0 0 1 1 0]);
  wires:
  "p{1}"/1 -> Delays 1/1("p{1}")
  Delays 1/1("pd{1,1}") -> "IW{1,1}"/1("pd{1,1}")
  "IW{1,1}"/1("iz{1,1}") -> netsum/1
  "b{1}"/1 -> netsum/2
  netsum/1 -> tansig/1
  tansig/1 -> "a{1}"/1
